simulink model "wlan_phy_rx_pmd"  (Simulink 7.8)
settings: Solver=VariableStepDiscrete  SolverMode=SingleTasking  StartTime=0.0  StopTime=simtime  RelTol=1e-3
PostLoadFcn:
  | wlan_phy_rx_init
InitFcn:
  | wlan_phy_rx_init
StartFcn:
  | wlan_phy_rx_init
values from neighbouring files (symbols this model uses):
  barker_seq20 = 0.95 * circshift(barker_seq20, [0 4]) ./ max(abs(barker_seq20))    [wlan_phy_rx_init.m]
  bit_scrambler_lfsr_bytes = bi2de(reshape(repmat(scr, 1, 8), 8, 127)', 'left-msb')    [wlan_phy_rx_init.m]
  deinterleave_ROM = [deinterleave_ROM interleave_64QAM zeros(1, 512-length(interleave_64QAM))]    [wlan_phy_rx_init.m]
  longCorr_coef_i = [3*real(LTS_corr)]    [wlan_phy_rx_init.m]
  longCorr_coef_q = [3*imag(LTS_corr)]    [wlan_phy_rx_init.m]
  MAX_CP_LEN = 32    [wlan_phy_rx_init.m]
  MAX_NCBPS = 288    [wlan_phy_rx_init.m]
  MAX_NUM_BYTES = 4096    [wlan_phy_rx_init.m]
  MAX_NUM_SC = 64    [wlan_phy_rx_init.m]
  MAX_NUM_SYMS = 600    [wlan_phy_rx_init.m]
  n_bits_preFFT_sampBuff = ceil(log2(4*MAX_NUM_SC))    [wlan_phy_rx_init.m]
  NCBPS_16QAM = 192    [wlan_phy_rx_init.m]
  NCBPS_64QAM = 288    [wlan_phy_rx_init.m]
  NCBPS_BPSK = 48    [wlan_phy_rx_init.m]
  NCBPS_QPSK = 96    [wlan_phy_rx_init.m]
  NDBPS_16QAM12 = 96    [wlan_phy_rx_init.m]
  NDBPS_16QAM34 = 144    [wlan_phy_rx_init.m]
  NDBPS_64QAM23 = 192    [wlan_phy_rx_init.m]
  NDBPS_64QAM34 = 216    [wlan_phy_rx_init.m]
  NDBPS_BPSK12 = 24    [wlan_phy_rx_init.m]
  NDBPS_BPSK34 = 36    [wlan_phy_rx_init.m]
  NDBPS_QPSK12 = 48    [wlan_phy_rx_init.m]
  NDBPS_QPSK34 = 72    [wlan_phy_rx_init.m]
  paylod_vec_samp_time = 8    [wlan_phy_rx_init.m]
  PHY_MIN_PKT_LEN = 14    [wlan_phy_rx_init.m]
  scr_ind_rev = zeros(1,128)    [wlan_phy_rx_init.m]
undefined symbols (not defined in the file or in its folder): gcbh, xlBlockLoadCallback, xlGetNormalizedPeriod, xlipgetpartsetting
libraries: xbsIndex_r4 x3119, simulink x1, xbsEDKLib_r4 x1
(part 9 of 19)

== system wlan_phy_rx_pmd/Detect & Decode/De-Interleave & De-Puncture/Rd Addr Gen ==
  note: This is a brute force de-interleaver- the ROM stores the
      | de-interleaving pattern for each mod scheme separately.
      | The 802.11 standard interleavers have enough structure that
      | a non-ROM implementation is possible. But this design will
      | enable other interleaving patterns without changes to logic.
  Block Wr Done : Inport
  mod_sel : Inport  Port=2
  Reset : Inport  Port=3
  rate_sel : Inport  Port=4
  Bus Creator : BusCreator  Inputs=2
  Bus Creator1 : BusCreator  Inputs=2
  Bus Creator2 : BusCreator  Inputs=2
  Ctrl : Subsystem
  De-Interleave DP-ROM : Subsystem
  Delay{1..4} (4 alike) : xbsIndex_r4/Delay  rst=off  infoeditControl=Selection of Reset will increase slice count due to use of real FFs and instead of SRLs  en=off  latency=1  dbl_ovrd=off  reg_retiming=off  block_type=delay
  Gateway Out{1..6,8..11} (10 alike) : xbsIndex_r4/Gateway Out  inherit_from_input=off  hdl_port=off  timing_constraint=None  locs_specified=off  LOCs={}  UseAsDAC=off  DACChannel='1'  block_type=gatewayout
  Int Rd Addr Gen : Scope  NumInputPorts=8
      settings: TimeRange=9000  YMin=0~0~0~0~-1~0~41.76470588235293~-0.1  YMax=2~1~1~1000~1~1~60.58823529411768~1.1  SaveName=ScopeData12
  Logical5 : xbsIndex_r4/Logical  logical_function=OR  inputs=2  en=off  latency=0  precision=Full  arith_type=Unsigned  n_bits=16  bin_pt=0  align_bp=on  dbl_ovrd=off  block_type=logical
  S-R Latch1 : Subsystem
  Rd Addr A : Outport
  Rd Addr B : Outport  Port=2
  Skip A : Outport  Port=3
  Skip B : Outport  Port=4
  Vout : Outport  Port=5
  wires:
  Ctrl/5(Vout) -> Delay2/1
  Gateway Out4/1 -> Int Rd Addr Gen/3  "Count En"
  Gateway Out3/1 -> Int Rd Addr Gen/2  "Wr done"
  Gateway Out2/1 -> Int Rd Addr Gen/1  "Rate Sel"
  Gateway Out8/1 -> Int Rd Addr Gen/6  "Vout"
  Logical5/1 -> S-R Latch1/2(R), Ctrl/3(Reset)
  Block Wr Done/1 -> Delay3/1
  S-R Latch1/1(Q) -> Ctrl/4(En), Gateway Out4/1
  Reset/1 -> Logical5/2
  Delay4/1 -> Skip A/1, Gateway Out9/1
  Delay3/1 -> S-R Latch1/1(S)
  Ctrl/6(Done) -> Logical5/1, Gateway Out3/1
  Delay1/1 -> Skip B/1, Gateway Out10/1
  De-Interleave DP-ROM/1(Addr A) -> Rd Addr A/1, Gateway Out6/1
  De-Interleave DP-ROM/2(Addr B) -> Rd Addr B/1, Gateway Out11/1
  Bus Creator1/1 -> Int Rd Addr Gen/7  "Rd Addr AB"
  Gateway Out6/1 -> Bus Creator1/1
  Gateway Out11/1 -> Bus Creator1/2
  mod_sel/1 -> Ctrl/1(mod_sel)
  rate_sel/1 -> Ctrl/2(rate_sel), Gateway Out2/1
  Ctrl/1(Ind A) -> De-Interleave DP-ROM/1(Ind A), Gateway Out1/1
  Ctrl/2(Ind B) -> De-Interleave DP-ROM/2(Ind B), Gateway Out5/1
  Ctrl/3(Skip A) -> Delay4/1
  Ctrl/4(Skip B) -> Delay1/1
  Delay2/1 -> Vout/1, Gateway Out8/1
  Gateway Out9/1 -> Bus Creator/1
  Gateway Out10/1 -> Bus Creator/2
  Bus Creator/1 -> Int Rd Addr Gen/8  "Skip AB"
  Bus Creator2/1 -> Int Rd Addr Gen/4  "Rd Ind AB"
  Gateway Out1/1 -> Bus Creator2/1
  Gateway Out5/1 -> Bus Creator2/2

== system wlan_phy_rx_pmd/Detect & Decode/De-Interleave & De-Puncture/Wr Addr Gen ==
  tvalid : Inport
  mod_sel : Inport  Port=2
  Reset : Inport  Port=3
  AddSub : xbsIndex_r4/AddSub  mode=Addition  use_carryin=off  use_carryout=off  en=off  latency=0  precision=User Defined  arith_type=Unsigned  n_bits=ceil(log2(MAX_NCBPS))  bin_pt=0  quantization=Truncate  overflow=Wrap  dbl_ovrd=off  use_behavioral_HDL=off  hw_selection=Fabric  pipelined=off  block_type=addsub
  Constant1 : xbsIndex_r4/Constant  const=NCBPS_QPSK-1  gui_display_data_type=Fixed-point  arith_type=Unsigned  n_bits=ceil(log2(MAX_NCBPS))  bin_pt=0  preci_type=Single  exp_width=8  frac_width=24  explicit_period=off  period=1  equ=P=C  opselect=C  inp2=PCIN>>17  opr=+  inp1=P  carry=CIN  dbl_ovrd=off  block_type=constant
  Constant3 : xbsIndex_r4/Constant  const=NCBPS_16QAM-1  gui_display_data_type=Fixed-point  arith_type=Unsigned  n_bits=ceil(log2(MAX_NCBPS))  bin_pt=0  preci_type=Single  exp_width=8  frac_width=24  explicit_period=off  period=1  equ=P=C  opselect=C  inp2=PCIN>>17  opr=+  inp1=P  carry=CIN  dbl_ovrd=off  block_type=constant
  Constant4 : xbsIndex_r4/Constant  const=NCBPS_64QAM-1  gui_display_data_type=Fixed-point  arith_type=Unsigned  n_bits=ceil(log2(MAX_NCBPS))  bin_pt=0  preci_type=Single  exp_width=8  frac_width=24  explicit_period=off  period=1  equ=P=C  opselect=C  inp2=PCIN>>17  opr=+  inp1=P  carry=CIN  dbl_ovrd=off  block_type=constant
  Constant5 : xbsIndex_r4/Constant  const=NCBPS_BPSK-1  gui_display_data_type=Fixed-point  arith_type=Unsigned  n_bits=ceil(log2(MAX_NCBPS))  bin_pt=0  preci_type=Single  exp_width=8  frac_width=24  explicit_period=off  period=1  equ=P=C  opselect=C  inp2=PCIN>>17  opr=+  inp1=P  carry=CIN  dbl_ovrd=off  block_type=constant
  Constant6 : xbsIndex_r4/Constant  const=1  gui_display_data_type=Fixed-point  arith_type=Unsigned  n_bits=1  bin_pt=0  preci_type=Single  exp_width=8  frac_width=24  explicit_period=off  period=1  equ=P=C  opselect=C  inp2=PCIN>>17  opr=+  inp1=P  carry=CIN  dbl_ovrd=off  block_type=constant
  Counter : xbsIndex_r4/Counter  cnt_type=Free Running  cnt_to=1  operation=Up  start_count=0  cnt_by_val=2  arith_type=Unsigned  n_bits=ceil(log2(MAX_NCBPS))  bin_pt=0  load_pin=off  rst=on  en=on  explicit_period=off  period=1  dbl_ovrd=off  use_behavioral_HDL=off  implementation=Fabric  block_type=counter
  DeInt Wr Addr : Scope  NumInputPorts=8
      settings: TimeRange=20000  YMin=0~0~0~0~0~-1~-1~-1  YMax=1~1~100~1~1~1~1~1  SaveName=ScopeData6
  Gateway Out{1..6} (6 alike) : xbsIndex_r4/Gateway Out  inherit_from_input=off  hdl_port=off  timing_constraint=None  locs_specified=off  LOCs={}  UseAsDAC=off  DACChannel='1'  block_type=gatewayout
  Logical5 : xbsIndex_r4/Logical  logical_function=OR  inputs=2  en=off  latency=0  precision=Full  arith_type=Unsigned  n_bits=16  bin_pt=0  align_bp=on  dbl_ovrd=off  block_type=logical
  Mux2 : xbsIndex_r4/Mux  inputs=4  en=off  latency=0  precision=Full  arith_type=Unsigned  n_bits=16  bin_pt=14  quantization=Truncate  overflow=Wrap  dbl_ovrd=off  block_type=mux
  Relational1 : xbsIndex_r4/Relational  mode=a>=b  en=off  latency=0  dbl_ovrd=off  block_type=relational
  Wr Addr A : Outport
  Wr Addr B : Outport  Port=2
  Wr En : Outport  Port=3
  Block Wr Done : Outport  Port=4
  wires:
  Mux2/1 -> Relational1/2
  Logical5/1 -> Counter/1, Gateway Out2/1
  Counter/1 -> Relational1/1, AddSub/2, Wr Addr A/1, Gateway Out4/1
  tvalid/1 -> Counter/2, Gateway Out3/1, Wr En/1, Gateway Out1/1
  Constant5/1 -> Mux2/2
  Constant1/1 -> Mux2/3
  Constant3/1 -> Mux2/4
  Constant4/1 -> Mux2/5
  mod_sel/1 -> Mux2/1
  Relational1/1 -> Gateway Out5/1, Block Wr Done/1, Logical5/2
  Reset/1 -> Logical5/1
  Gateway Out2/1 -> DeInt Wr Addr/1  "Reset"
  Gateway Out3/1 -> DeInt Wr Addr/2  "Valid In"
  Gateway Out4/1 -> DeInt Wr Addr/3  "Wr Addr A"
  Gateway Out1/1 -> DeInt Wr Addr/5  "Wr En"
  Gateway Out5/1 -> DeInt Wr Addr/6  "Block Done"
  Constant6/1 -> AddSub/1
  AddSub/1 -> Wr Addr B/1, Gateway Out6/1
  Gateway Out6/1 -> DeInt Wr Addr/4  "Wr Addr B"

== system wlan_phy_rx_pmd/Detect & Decode/Decode/Byte Count ==
  Byte Valid : Inport
  Decode Start : Inport  Port=2
  Last Byte Ind : Inport  Port=3
  Byte Counter : xbsIndex_r4/Counter  cnt_type=Free Running  cnt_to=1  operation=Up  start_count=0  cnt_by_val=1  arith_type=Unsigned  n_bits=14  bin_pt=0  load_pin=off  rst=on  en=on  explicit_period=off  period=1  dbl_ovrd=off  use_behavioral_HDL=off  implementation=Fabric  block_type=counter
  Inverter1 : xbsIndex_r4/Inverter  en=off  latency=0  dbl_ovrd=off  block_type=inv
  Logical : xbsIndex_r4/Logical  logical_function=AND  inputs=2  en=off  latency=0  precision=Full  arith_type=Unsigned  n_bits=16  bin_pt=0  align_bp=on  dbl_ovrd=off  block_type=logical
  Logical1 : xbsIndex_r4/Logical  logical_function=OR  inputs=2  en=off  latency=0  precision=Full  arith_type=Unsigned  n_bits=16  bin_pt=0  align_bp=on  dbl_ovrd=off  block_type=logical
  Logical3 : xbsIndex_r4/Logical  logical_function=AND  inputs=2  en=off  latency=0  precision=Full  arith_type=Unsigned  n_bits=16  bin_pt=0  align_bp=on  dbl_ovrd=off  block_type=logical
  Relational2 : xbsIndex_r4/Relational  mode=a>=b  en=off  latency=0  dbl_ovrd=off  block_type=relational
  S-R Latch1 : Subsystem
  " Byte Valid" : Outport
  Byte Index : Outport  Port=2
  Last Byte : Outport  Port=3
  wires:
  Logical/1 -> Byte Counter/2, " Byte Valid"/1
  Byte Counter/1 -> Byte Index/1, Relational2/1
  Logical3/1 -> S-R Latch1/2(R), Last Byte/1
  Decode Start/1 -> S-R Latch1/1(S), Logical1/1
  S-R Latch1/1(Q) -> Logical/1, Inverter1/1
  Byte Valid/1 -> Logical/2, Logical3/1
  Relational2/1 -> Logical3/2
  Last Byte Ind/1 -> Relational2/2
  Inverter1/1 -> Logical1/2
  Logical1/1 -> Byte Counter/1

== system wlan_phy_rx_pmd/Detect & Decode/Decode/Ctrl ==
  note: early_trace input requires pulse coincident
      | with last vin for early bits, staying asserted
      | until at least first decoder vout.
  note: 22: (NUM_SIGNAL_BYTES * 8) - 2
  dec_vin : Inport
  Reset : Inport  Port=2
  Start : Inport  Port=3
  Num Bytes : Inport  Port=4
  dec_vout : Inport  Port=5
  AddSub : xbsIndex_r4/AddSub  mode=Addition  use_carryin=off  use_carryout=off  en=off  latency=0  precision=Full  arith_type=Unsigned  n_bits=16  bin_pt=14  quantization=Truncate  overflow=Wrap  dbl_ovrd=off  use_behavioral_HDL=off  hw_selection=Fabric  pipelined=off  block_type=addsub
  Coded Bit Counter : xbsIndex_r4/Counter  cnt_type=Free Running  cnt_to=1  operation=Up  start_count=0  cnt_by_val=1  arith_type=Unsigned  n_bits=2+ceil(log2(MAX_NUM_BYTES))  bin_pt=0  load_pin=off  rst=on  en=on  explicit_period=off  period=1  dbl_ovrd=off  use_behavioral_HDL=off  implementation=Fabric  block_type=counter
  Concat : xbsIndex_r4/Concat  num_inputs=2  dbl_ovrd=off  block_type=concat
  Constant1 : xbsIndex_r4/Constant  const=14  gui_display_data_type=Fixed-point  arith_type=Unsigned  n_bits=5  bin_pt=0  preci_type=Single  exp_width=8  frac_width=24  explicit_period=off  period=1  equ=P=C  opselect=C  inp2=PCIN>>17  opr=+  inp1=P  carry=CIN  dbl_ovrd=off  block_type=constant
  Constant2 : xbsIndex_r4/Constant  const=0  gui_display_data_type=Fixed-point  arith_type=Unsigned  n_bits=3  bin_pt=0  preci_type=Single  exp_width=8  frac_width=24  explicit_period=off  period=1  equ=P=C  opselect=C  inp2=PCIN>>17  opr=+  inp1=P  carry=CIN  dbl_ovrd=off  block_type=constant
  Constant3 : xbsIndex_r4/Constant  const=3*8 - 2  gui_display_data_type=Fixed-point  arith_type=Unsigned  n_bits=5  bin_pt=0  preci_type=Single  exp_width=8  frac_width=24  explicit_period=off  period=1  equ=P=C  opselect=C  inp2=PCIN>>17  opr=+  inp1=P  carry=CIN  dbl_ovrd=off  block_type=constant
  Convert{,1..2,7} (4 alike) : xbsIndex_r4/Convert  gui_display_data_type=Fixed-point  arith_type=Unsigned  n_bits=1  bin_pt=0  float_type=Single  exp_bits=8  fraction_bits=24  quantization=Truncate  overflow=Wrap  en=off  latency=0  dbl_ovrd=off  pipeline=off  block_type=convert
  Decoder Ctrl : Scope  NumInputPorts=8
      settings: TimeRange=10000  YMin=0~0~0~0~0~0~0~0  YMax=1~1~100~1~1000~1~1~1  SaveName=ScopeData40
  Delay1 : xbsIndex_r4/Delay  rst=off  infoeditControl=Selection of Reset will increase slice count due to use of real FFs and instead of SRLs  en=off  latency=2  dbl_ovrd=off  reg_retiming=off  block_type=delay
  Delay2 : xbsIndex_r4/Delay  rst=off  infoeditControl=Selection of Reset will increase slice count due to use of real FFs and instead of SRLs  en=off  latency=2  dbl_ovrd=off  reg_retiming=off  block_type=delay
  Gateway Out{1..5,7..8,10} (8 alike) : xbsIndex_r4/Gateway Out  inherit_from_input=off  hdl_port=off  timing_constraint=None  locs_specified=off  LOCs={}  UseAsDAC=off  DACChannel='1'  block_type=gatewayout
  Inverter : xbsIndex_r4/Inverter  en=off  latency=0  dbl_ovrd=off  block_type=inv
  Logical1 : xbsIndex_r4/Logical  logical_function=AND  inputs=2  en=off  latency=0  precision=Full  arith_type=Unsigned  n_bits=16  bin_pt=0  align_bp=on  dbl_ovrd=off  block_type=logical
  Logical3 : xbsIndex_r4/Logical  logical_function=OR  inputs=2  en=off  latency=0  precision=Full  arith_type=Unsigned  n_bits=16  bin_pt=0  align_bp=on  dbl_ovrd=off  block_type=logical
  Relational2 : xbsIndex_r4/Relational  mode=a<b  en=off  latency=0  dbl_ovrd=off  block_type=relational
  Relational3 : xbsIndex_r4/Relational  mode=a=b  en=off  latency=0  dbl_ovrd=off  block_type=relational
  S-R Latch1 : Subsystem
  negedge : Subsystem
  vin : Outport
  rst# : Outport  Port=2
  start : Outport  Port=3
  pkt_end : Outport  Port=4
  early_trace_en : Outport  Port=5
  wires:
  dec_vin/1 -> Coded Bit Counter/2, Gateway Out3/1, Logical1/2
  dec_vout/1 -> S-R Latch1/2(R)
  S-R Latch1/1(Q) -> Convert1/1
  Start/1 -> Coded Bit Counter/1, Gateway Out1/1, Logical3/2, Delay1/1
  Coded Bit Counter/1 -> Gateway Out4/1, Relational3/2, Relational2/1
  Num Bytes/1 -> Concat/1, Gateway Out2/1
  Constant2/1 -> Concat/2
  Concat/1 -> AddSub/1
  Inverter/1 -> Convert/1
  Logical3/1 -> Inverter/1
  Reset/1 -> Logical3/1, Gateway Out10/1
  Delay1/1 -> Convert7/1
  Convert/1 -> rst#/1
  Convert7/1 -> start/1
  Relational3/1 -> S-R Latch1/1(S)
  Convert1/1 -> early_trace_en/1, Gateway Out5/1
  Gateway Out10/1 -> Decoder Ctrl/1  "Reset"
  Gateway Out1/1 -> Decoder Ctrl/2  "Start"
  Gateway Out2/1 -> Decoder Ctrl/3  "Num Bytes"
  Gateway Out3/1 -> Decoder Ctrl/4  "Vin in"
  Gateway Out4/1 -> Decoder Ctrl/5  "Bit Counter"
  Gateway Out5/1 -> Decoder Ctrl/6  "Early Trace En"
  negedge/1(Q) -> Delay2/1
  Gateway Out7/1 -> Decoder Ctrl/7  "End"
  Constant3/1 -> Relational3/1
  Logical1/1 -> Convert2/1
  Convert2/1 -> vin/1, Gateway Out8/1
  Gateway Out8/1 -> Decoder Ctrl/8  "Vin Out"
  Relational2/1 -> Logical1/1, negedge/1(D)
  Constant1/1 -> AddSub/2
  AddSub/1 -> Relational2/2
  Delay2/1 -> pkt_end/1, Gateway Out7/1

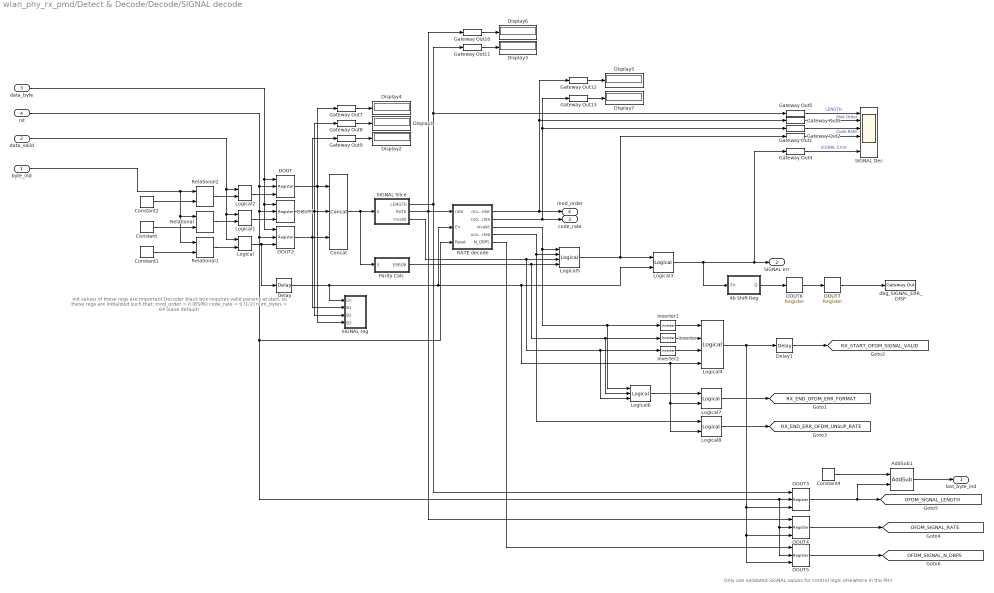
== system wlan_phy_rx_pmd/Detect & Decode/Decode/SIGNAL decode  ==
  note: Init values of these regs are important
      | Decoder black box requires valid params at start,
      | so these regs are initialzied such that:
      | mod_order = 0 (BSPK)
      | code_rate = 0 (1/2)
      | num_bytes = 64 (sane default)
  note: Only use validated SIGNAL values for
      | control logic elsewhere in the PHY
  byte_ind : Inport
  data_valid : Inport  Port=2
  data_byte : Inport  Port=3
  rst : Inport  Port=4
  4b Shift Reg : Subsystem
  AddSub1 : xbsIndex_r4/AddSub  mode=Addition  use_carryin=off  use_carryout=off  en=off  latency=0  precision=User Defined  arith_type=Unsigned  n_bits=12  bin_pt=0  quantization=Truncate  overflow=Wrap  dbl_ovrd=off  use_behavioral_HDL=off  hw_selection=Fabric  pipelined=off  block_type=addsub
  Concat : xbsIndex_r4/Concat  num_inputs=3  dbl_ovrd=off  block_type=concat
  Constant : xbsIndex_r4/Constant  const=1  gui_display_data_type=Fixed-point  arith_type=Unsigned  n_bits=2  bin_pt=0  preci_type=Single  exp_width=8  frac_width=24  explicit_period=off  period=1  equ=P=C  opselect=C  inp2=PCIN>>17  opr=+  inp1=P  carry=CIN  dbl_ovrd=off  block_type=constant
  Constant1 : xbsIndex_r4/Constant  const=2  gui_display_data_type=Fixed-point  arith_type=Unsigned  n_bits=2  bin_pt=0  preci_type=Single  exp_width=8  frac_width=24  explicit_period=off  period=1  equ=P=C  opselect=C  inp2=PCIN>>17  opr=+  inp1=P  carry=CIN  dbl_ovrd=off  block_type=constant
  Constant2 : xbsIndex_r4/Constant  const=0  gui_display_data_type=Fixed-point  arith_type=Unsigned  n_bits=2  bin_pt=0  preci_type=Single  exp_width=8  frac_width=24  explicit_period=off  period=1  equ=P=C  opselect=C  inp2=PCIN>>17  opr=+  inp1=P  carry=CIN  dbl_ovrd=off  block_type=constant
  Constant4 : xbsIndex_r4/Constant  const=4  gui_display_data_type=Fixed-point  arith_type=Unsigned  n_bits=3  bin_pt=0  preci_type=Single  exp_width=8  frac_width=24  explicit_period=off  period=1  equ=P=C  opselect=C  inp2=PCIN>>17  opr=+  inp1=P  carry=CIN  dbl_ovrd=off  block_type=constant
  DOUT : xbsIndex_r4/Register  init=hex2dec('D6')  rst=on  en=on  dbl_ovrd=off  block_type=register
  DOUT1 : xbsIndex_r4/Register  init=hex2dec('E0')  rst=on  en=on  dbl_ovrd=off  block_type=register
  DOUT2 : xbsIndex_r4/Register  init=hex2dec('00')  rst=on  en=on  dbl_ovrd=off  block_type=register
  DOUT3 : xbsIndex_r4/Register  init=10  rst=on  en=on  dbl_ovrd=off  block_type=register
  DOUT4 : xbsIndex_r4/Register  init=11  rst=on  en=on  dbl_ovrd=off  block_type=register
  DOUT5 : xbsIndex_r4/Register  init=24  rst=on  en=on  dbl_ovrd=off  block_type=register
  DOUT6 : xbsIndex_r4/Register  init=0  rst=off  en=off  dbl_ovrd=off  block_type=register
  DOUT7 : xbsIndex_r4/Register  init=0  rst=off  en=off  dbl_ovrd=off  block_type=register
  Delay : xbsIndex_r4/Delay  rst=off  infoeditControl=Selection of Reset will increase slice count due to use of real FFs and instead of SRLs  en=off  latency=1  dbl_ovrd=off  reg_retiming=off  block_type=delay
  Delay1 : xbsIndex_r4/Delay  rst=off  infoeditControl=Selection of Reset will increase slice count due to use of real FFs and instead of SRLs  en=off  latency=1  dbl_ovrd=off  reg_retiming=off  block_type=delay
  Display{1..7} (7 alike) : Display
  Gateway Out{1..2,4..13} (12 alike) : xbsIndex_r4/Gateway Out  inherit_from_input=off  hdl_port=off  timing_constraint=None  locs_specified=off  LOCs={}  UseAsDAC=off  DACChannel='1'  block_type=gatewayout
  Inverter : xbsIndex_r4/Inverter  en=off  latency=0  dbl_ovrd=off  block_type=inv
  Inverter1 : xbsIndex_r4/Inverter  en=off  latency=0  dbl_ovrd=off  block_type=inv
  Inverter2 : xbsIndex_r4/Inverter  en=off  latency=0  dbl_ovrd=off  block_type=inv
  Logical{,1..3,7..8} (6 alike) : xbsIndex_r4/Logical  logical_function=AND  inputs=2  en=off  latency=0  precision=Full  arith_type=Unsigned  n_bits=16  bin_pt=0  align_bp=on  dbl_ovrd=off  block_type=logical
  Logical4 : xbsIndex_r4/Logical  logical_function=AND  inputs=4  en=off  latency=0  precision=Full  arith_type=Unsigned  n_bits=16  bin_pt=0  align_bp=on  dbl_ovrd=off  block_type=logical
  Logical5 : xbsIndex_r4/Logical  logical_function=OR  inputs=4  en=off  latency=0  precision=Full  arith_type=Unsigned  n_bits=16  bin_pt=0  align_bp=on  dbl_ovrd=off  block_type=logical
  Logical6 : xbsIndex_r4/Logical  logical_function=OR  inputs=3  en=off  latency=0  precision=Full  arith_type=Unsigned  n_bits=16  bin_pt=0  align_bp=on  dbl_ovrd=off  block_type=logical
  Parity Calc : Subsystem
  RATE decode : Subsystem
  Relational : xbsIndex_r4/Relational  mode=a=b  en=off  latency=0  dbl_ovrd=off  block_type=relational
  Relational1 : xbsIndex_r4/Relational  mode=a=b  en=off  latency=0  dbl_ovrd=off  block_type=relational
  Relational2 : xbsIndex_r4/Relational  mode=a=b  en=off  latency=0  dbl_ovrd=off  block_type=relational
  SIGNAL Dec : Scope  NumInputPorts=6
      settings: TimeRange=25000  YMin=60.8~0.95~-1~0.95~-1~-1  YMax=67.2~1.05~1~1.05~1~1  SaveName=ScopeData18
  SIGNAL Slice : Subsystem
  SIGNAL reg : Subsystem
  dbg_SIGNAL_ERR_DISP : xbsIndex_r4/Gateway Out  inherit_from_input=off  hdl_port=on  timing_constraint=None  locs_specified=off  LOCs={}  UseAsDAC=off  DACChannel='1'  block_type=gatewayout
  last_byte_ind : Outport
  SIGNAL err : Outport  Port=2
  code_rate : Outport  Port=3
  mod_order : Outport  Port=4
  tag scope: OFDM_SIGNAL_LENGTH=global, OFDM_SIGNAL_N_DBPS=global, OFDM_SIGNAL_RATE=global, RX_END_ERR_OFDM_UNSUP_RATE=global, RX_END_OFDM_ERR_FORMAT=global, RX_START_OFDM_SIGNAL_VALID=global
  wires:
  data_valid/1 -> Logical2/1, Logical1/1, Logical/1
  byte_ind/1 -> Relational2/1, Relational1/1, Relational/1
  data_byte/1 -> DOUT/1, DOUT1/1, DOUT2/1
  Gateway Out5/1 -> SIGNAL Dec/1  "LENGTH"
  Gateway Out6/1 -> SIGNAL Dec/2  "Mod Order"
  Gateway Out1/1 -> SIGNAL Dec/3  "Code Rate"
  Gateway Out2/1 -> SIGNAL Dec/4
  Gateway Out4/1 -> SIGNAL Dec/6  "SIGNAL Error"
  Constant/1 -> Relational/2
  Constant1/1 -> Relational1/2
  Constant2/1 -> Relational2/2
  Relational1/1 -> Logical/2
  Relational2/1 -> Logical2/2
  Relational/1 -> Logical1/2
  Logical/1 -> Delay/1, DOUT2/3
  Concat/1 -> SIGNAL Slice/1(S), Parity Calc/1(S)
  SIGNAL Slice/2(RATE) -> Gateway Out10/1, DOUT4/1, RATE decode/1(rate)
  SIGNAL Slice/1(LENGTH) -> DOUT3/1, Gateway Out11/1, Gateway Out5/1
  Parity Calc/1(ERROR) -> Logical5/4, Inverter/1, Logical6/2
  Logical5/1 -> Logical3/1, Gateway Out2/1
  DOUT/1 -> Concat/1, Gateway Out7/1, SIGNAL reg/4(B3)
  DOUT1/1 -> Concat/2, Gateway Out8/1, SIGNAL reg/3(B2)
  DOUT2/1 -> Concat/3, Gateway Out9/1, SIGNAL reg/2(B1)
  Logical2/1 -> DOUT/3
  Logical1/1 -> DOUT1/3
  rst/1 -> DOUT/2, DOUT1/2, DOUT2/2, DOUT3/2, DOUT4/2, DOUT5/2, RATE decode/3(Reset)
  RATE decode/1(mod_order) -> mod_order/1, Gateway Out6/1, Gateway Out12/1
  RATE decode/2(code_rate) -> code_rate/1, Gateway Out1/1, Gateway Out13/1
  Delay/1 -> SIGNAL reg/1(En), Logical3/2, Logical4/4, Logical7/2, Logical8/2, RATE decode/2(En)
  Gateway Out7/1 -> Display4/1
  Gateway Out8/1 -> Display1/1
  Gateway Out9/1 -> Display2/1
  Gateway Out10/1 -> Display6/1
  Gateway Out11/1 -> Display3/1
  Gateway Out12/1 -> Display5/1
  Gateway Out13/1 -> Display7/1
  Logical3/1 -> SIGNAL err/1, Gateway Out4/1, 4b Shift Reg/1(En)
  Inverter/1 -> Logical4/2
  Logical4/1 -> Delay1/1, DOUT3/3, DOUT5/3, DOUT4/3
  SIGNAL Slice/3(invalid) -> Logical5/3, Inverter2/1, Logical6/3
  RATE decode/4(unsupported) -> Logical5/2, Logical8/1
  RATE decode/3(invalid) -> Logical5/1, Inverter1/1, Logical6/1
  Inverter1/1 -> Logical4/1
  Inverter2/1 -> Logical4/3
  Logical6/1 -> Logical7/1
  Logical7/1 -> [RX_END_OFDM_ERR_FORMAT]
  Logical8/1 -> [RX_END_ERR_OFDM_UNSUP_RATE]
  DOUT4/1 -> [OFDM_SIGNAL_RATE]
  DOUT3/1 -> [OFDM_SIGNAL_LENGTH], AddSub1/2
  Delay1/1 -> [RX_START_OFDM_SIGNAL_VALID]
  AddSub1/1 -> last_byte_ind/1
  RATE decode/5(N_DBPS) -> DOUT5/1
  DOUT5/1 -> [OFDM_SIGNAL_N_DBPS]
  Constant4/1 -> AddSub1/1
  DOUT6/1 -> DOUT7/1
  DOUT7/1 -> dbg_SIGNAL_ERR_DISP/1
  4b Shift Reg/1(Q) -> DOUT6/1

== system wlan_phy_rx_pmd/Detect & Decode/Decode/Safe ToUint8 ==
  note: Simulation-only block: Sets output to 0
      | if input is indeterminate, which occurs at
      | the blackbox outputs before the first reset
  A : Inport
  Convert1 : xbsIndex_r4/Convert  gui_display_data_type=Fixed-point  arith_type=Unsigned  n_bits=8  bin_pt=0  float_type=Single  exp_bits=8  fraction_bits=24  quantization=Truncate  overflow=Wrap  en=off  latency=0  dbl_ovrd=off  pipeline=off  block_type=convert
  Indet Check : Subsystem
  Simulation Multiplexer : xbsIndex_r4/Simulation Multiplexer  sim_sel=1  hw_sel=2  block_type=simmux
      settings: infoedit="Distinguish input subsystems as \"simulation only\" and \"generation only\".  The input specified For Simulation will be used during Simulink simulation.  The input specified For Generation will be used during code generation.  This block will typically be used on the output of a HW Co-Simulation, Black Box, or ModelSim subsystem.<br><br>Hardware Notes: This block costs nothing."
  B : Outport
  wires:
  A/1 -> Indet Check/1(In1), Simulation Multiplexer/2
  Indet Check/1(Out1) -> Simulation Multiplexer/1
  Simulation Multiplexer/1 -> Convert1/1
  Convert1/1 -> B/1

== system wlan_phy_rx_pmd/Detect & Decode/Decode/Stretch ==
  D : Inport
  Delay1 : xbsIndex_r4/Delay  rst=off  infoeditControl=Selection of Reset will increase slice count due to use of real FFs and instead of SRLs  en=off  latency=1  dbl_ovrd=off  reg_retiming=off  block_type=delay
  Delay4 : xbsIndex_r4/Delay  rst=off  infoeditControl=Selection of Reset will increase slice count due to use of real FFs and instead of SRLs  en=off  latency=1  dbl_ovrd=off  reg_retiming=off  block_type=delay
  Delay5 : xbsIndex_r4/Delay  rst=off  infoeditControl=Selection of Reset will increase slice count due to use of real FFs and instead of SRLs  en=off  latency=1  dbl_ovrd=off  reg_retiming=off  block_type=delay
  Logical4 : xbsIndex_r4/Logical  logical_function=OR  inputs=4  en=off  latency=0  precision=Full  arith_type=Unsigned  n_bits=16  bin_pt=0  align_bp=on  dbl_ovrd=off  block_type=logical
  Q : Outport
  wires:
  D/1 -> Logical4/1, Delay1/1
  Delay1/1 -> Logical4/2, Delay4/1
  Delay4/1 -> Logical4/3, Delay5/1
  Delay5/1 -> Logical4/4
  Logical4/1 -> Q/1

== system wlan_phy_rx_pmd/Detect & Decode/Descramble/Descram Byte Calc ==
  note: SERVICE field is used to lookup initial state
      | of Tx scrambler. This state becomes the starting
      | index to the bytewise descrambler logic. This
      | block is a parallel version of the bitwise scrambling
      | specified in the 802.11 spec.
  note: Don't keep the reset signal asserted if a packet
      | happens to end on byte 126 when the next
      | SERVICE field arrives. Without this not/and
      | every 126th packet will be descrambled wrong
      | (when all pkts have the same length)
  data_byte : Inport
  data_valid : Inport  Port=2
  data_ind : Inport  Port=3
  3LSB1 : xbsIndex_r4/Slice  nbits=3  boolean_output=off  mode=Lower Bit Location + Width  bit1=0  base1=MSB of Input  bit0=0  base0=LSB of Input  dbl_ovrd=off  block_type=slice
  4MSB1 : xbsIndex_r4/Slice  nbits=4  boolean_output=off  mode=Upper Bit Location + Width  bit1=0  base1=MSB of Input  bit0=0  base0=LSB of Input  dbl_ovrd=off  block_type=slice
  Concat1 : xbsIndex_r4/Concat  num_inputs=2  dbl_ovrd=off  block_type=concat
  Constant : xbsIndex_r4/Constant  const=3  gui_display_data_type=Fixed-point  arith_type=Unsigned  n_bits=4  bin_pt=0  preci_type=Single  exp_width=8  frac_width=24  explicit_period=off  period=1  equ=P=C  opselect=C  inp2=PCIN>>17  opr=+  inp1=P  carry=CIN  dbl_ovrd=off  block_type=constant
  Constant1 : xbsIndex_r4/Constant  const=126  gui_display_data_type=Fixed-point  arith_type=Unsigned  n_bits=7  bin_pt=0  preci_type=Single  exp_width=8  frac_width=24  explicit_period=off  period=1  equ=P=C  opselect=C  inp2=PCIN>>17  opr=+  inp1=P  carry=CIN  dbl_ovrd=off  block_type=constant
  Constant2 : xbsIndex_r4/Constant  const=0  gui_display_data_type=Fixed-point  arith_type=Unsigned  n_bits=8  bin_pt=0  preci_type=Single  exp_width=8  frac_width=24  explicit_period=off  period=1  equ=P=C  opselect=C  inp2=PCIN>>17  opr=+  inp1=P  carry=CIN  dbl_ovrd=off  block_type=constant
  Counter : xbsIndex_r4/Counter  cnt_type=Free Running  cnt_to=126  operation=Up  start_count=0  cnt_by_val=1  arith_type=Unsigned  n_bits=7  bin_pt=0  load_pin=on  rst=on  en=on  explicit_period=off  period=1  dbl_ovrd=off  use_behavioral_HDL=off  implementation=Fabric  block_type=counter
  Delay{1..4} (4 alike) : xbsIndex_r4/Delay  rst=off  infoeditControl=Selection of Reset will increase slice count due to use of real FFs and instead of SRLs  en=off  latency=1  dbl_ovrd=off  reg_retiming=off  block_type=delay
  Delay5 : xbsIndex_r4/Delay  rst=off  infoeditControl=Selection of Reset will increase slice count due to use of real FFs and instead of SRLs  en=off  latency=3  dbl_ovrd=off  reg_retiming=off  block_type=delay
  Descrm Ind : Scope  NumInputPorts=8
      settings: TimeRange=8000  YMin=70~102~50~-1~-5~-5~-5~-5  YMax=210~105~135~1~5~5~5~5  SaveName=ScopeData19
  Gateway Out{1..8} (8 alike) : xbsIndex_r4/Gateway Out  inherit_from_input=off  hdl_port=off  timing_constraint=None  locs_specified=off  LOCs={}  UseAsDAC=off  DACChannel='1'  block_type=gatewayout
  Inverter : xbsIndex_r4/Inverter  en=off  latency=0  dbl_ovrd=off  block_type=inv
  LFSR Init : Subsystem
  LFSR Init State LUT : xbsIndex_r4/ROM  depth=length(scr_ind_rev)  initVector=scr_ind_rev  distributed_mem=Distributed memory  rst=off  init_reg=0  en=off  latency=0  gui_display_data_type=Fixed-point  arith_type=Unsigned  n_bits=7  bin_pt=0  preci_type=Single  exp_width=8  frac_width=24  dbl_ovrd=off  optimize=Area  use_rpm=on  block_type=sprom
  LFSR by Byte : xbsIndex_r4/ROM  depth=length(bit_scrambler_lfsr_bytes)  initVector=bit_scrambler_lfsr_bytes  distributed_mem=Block RAM  rst=off  init_reg=0  en=off  latency=1  gui_display_data_type=Fixed-point  arith_type=Unsigned  n_bits=8  bin_pt=0  preci_type=Single  exp_width=8  frac_width=24  dbl_ovrd=off  optimize=Area  use_rpm=on  block_type=sprom
  Logical : xbsIndex_r4/Logical  logical_function=AND  inputs=2  en=off  latency=0  precision=Full  arith_type=Unsigned  n_bits=16  bin_pt=0  align_bp=on  dbl_ovrd=off  block_type=logical
  Logical1 : xbsIndex_r4/Logical  logical_function=OR  inputs=2  en=off  latency=0  precision=Full  arith_type=Unsigned  n_bits=16  bin_pt=0  align_bp=on  dbl_ovrd=off  block_type=logical
  Logical2 : xbsIndex_r4/Logical  logical_function=AND  inputs=3  en=off  latency=0  precision=Full  arith_type=Unsigned  n_bits=16  bin_pt=0  align_bp=on  dbl_ovrd=off  block_type=logical
  Mux : xbsIndex_r4/Mux  inputs=2  en=off  latency=0  precision=Full  arith_type=Unsigned  n_bits=16  bin_pt=14  quantization=Truncate  overflow=Wrap  dbl_ovrd=off  block_type=mux
  Relational : xbsIndex_r4/Relational  mode=a=b  en=off  latency=0  dbl_ovrd=off  block_type=relational
  Relational1 : xbsIndex_r4/Relational  mode=a=b  en=off  latency=0  dbl_ovrd=off  block_type=relational
  Relational2 : xbsIndex_r4/Relational  mode=a>=b  en=off  latency=0  dbl_ovrd=off  block_type=relational
  SERVICE : xbsIndex_r4/Register  init=0  rst=off  en=on  dbl_ovrd=off  block_type=register
  Descram Val : Outport
  wires:
  Logical2/1 -> Counter/3
  Relational1/1 -> Logical2/1
  Delay4/1 -> Gateway Out3/1
  Delay2/1 -> Delay4/1
  Concat1/1 -> Gateway Out1/1, Counter/2
  Gateway Out4/1 -> Descrm Ind/6
  4MSB1/1 -> Concat1/2
  3LSB1/1 -> Concat1/1
  Gateway Out3/1 -> Descrm Ind/5
  Gateway Out2/1 -> Descrm Ind/4
  Gateway Out1/1 -> Descrm Ind/3
  Gateway Out6/1 -> Descrm Ind/2
  Gateway Out5/1 -> Descrm Ind/1
  Counter/1 -> LFSR by Byte/1, Gateway Out2/1, Relational1/1
  Constant1/1 -> Relational1/2
  Delay1/1 -> Logical2/2, Logical1/2, Delay2/1
  Logical1/1 -> Counter/4
  Delay3/1 -> Logical1/1, Counter/1, Inverter/1
  LFSR Init State LUT/1 -> 4MSB1/1, 3LSB1/1, Gateway Out6/1
  LFSR by Byte/1 -> Gateway Out4/1, Mux/3
  data_ind/1 -> Relational/1, Relational2/1, Gateway Out8/1
  data_valid/1 -> Delay1/1, Logical/1
  data_byte/1 -> SERVICE/1
  LFSR Init/1(I) -> Gateway Out5/1, LFSR Init State LUT/1
  SERVICE/1 -> LFSR Init/1(B)
  Logical/1 -> Delay3/1, SERVICE/2
  Relational/1 -> Logical/2
  Constant/1 -> Relational/2, Relational2/2
  Mux/1 -> Descram Val/1
  Relational2/1 -> Delay5/1
  Constant2/1 -> Mux/2
  Gateway Out7/1 -> Descrm Ind/7
  Gateway Out8/1 -> Descrm Ind/8
  Delay5/1 -> Mux/1, Gateway Out7/1
  Inverter/1 -> Logical2/3

== system wlan_phy_rx_pmd/Detect & Decode/Descramble/Endian Swap ==
  B : Inport
  Concat : xbsIndex_r4/Concat  num_inputs=8  dbl_ovrd=off  block_type=concat
  b0 : xbsIndex_r4/Slice  nbits=1  boolean_output=off  mode=Lower Bit Location + Width  bit1=0  base1=MSB of Input  bit0=0  base0=LSB of Input  dbl_ovrd=off  block_type=slice
  b1 : xbsIndex_r4/Slice  nbits=1  boolean_output=off  mode=Lower Bit Location + Width  bit1=0  base1=MSB of Input  bit0=1  base0=LSB of Input  dbl_ovrd=off  block_type=slice
  b2 : xbsIndex_r4/Slice  nbits=1  boolean_output=off  mode=Lower Bit Location + Width  bit1=0  base1=MSB of Input  bit0=2  base0=LSB of Input  dbl_ovrd=off  block_type=slice
  b3 : xbsIndex_r4/Slice  nbits=1  boolean_output=off  mode=Lower Bit Location + Width  bit1=0  base1=MSB of Input  bit0=3  base0=LSB of Input  dbl_ovrd=off  block_type=slice
  b4 : xbsIndex_r4/Slice  nbits=1  boolean_output=off  mode=Lower Bit Location + Width  bit1=0  base1=MSB of Input  bit0=4  base0=LSB of Input  dbl_ovrd=off  block_type=slice
  b5 : xbsIndex_r4/Slice  nbits=1  boolean_output=off  mode=Lower Bit Location + Width  bit1=0  base1=MSB of Input  bit0=5  base0=LSB of Input  dbl_ovrd=off  block_type=slice
  b6 : xbsIndex_r4/Slice  nbits=1  boolean_output=off  mode=Lower Bit Location + Width  bit1=0  base1=MSB of Input  bit0=6  base0=LSB of Input  dbl_ovrd=off  block_type=slice
  b7 : xbsIndex_r4/Slice  nbits=1  boolean_output=off  mode=Lower Bit Location + Width  bit1=0  base1=MSB of Input  bit0=7  base0=LSB of Input  dbl_ovrd=off  block_type=slice
  I : Outport
  wires:
  b7/1 -> Concat/8
  Concat/1 -> I/1
  b6/1 -> Concat/7
  b5/1 -> Concat/6
  b4/1 -> Concat/5
  b3/1 -> Concat/4
  b2/1 -> Concat/3
  b1/1 -> Concat/2
  b0/1 -> Concat/1
  B/1 -> b7/1, b6/1, b5/1, b4/1, b3/1, b2/1, b1/1, b0/1

== system wlan_phy_rx_pmd/Detect & Decode/Soft Demap/16QAM Soft De-Map ==
  note: 16-QAM Demap:
      | Each sym is [b0 b1 b2 b3]
      | b0 = 1 if (I > 0)
      | b1 = 1 if(abs(I) < 2/sqrt(10))
      | b2 = 1 if(Q > 0)
      | b3 = 1 if(abs(Q) < 2/sqrt(10))
  IQ Valid : Inport
  I : Inport  Port=2
  Q : Inport  Port=3
  Reest : Inport  Port=4
  Absolute : xbsIndex_r4/Absolute  precision=Full  arith_type=Unsigned  n_bits=8  bin_pt=0  quantization=Truncate  overflow=Saturate  en=off  latency=0  dbl_ovrd=off  block_type=abs
  AddSub : xbsIndex_r4/AddSub  mode=Subtraction  use_carryin=off  use_carryout=off  en=off  latency=0  precision=User Defined  arith_type="Signed  (2's comp)"  n_bits=8  bin_pt=7  quantization=Truncate  overflow=Saturate  dbl_ovrd=off  use_behavioral_HDL=off  hw_selection=Fabric  pipelined=off  block_type=addsub
  Constant : xbsIndex_r4/Constant  const=2/sqrt(10)  gui_display_data_type=Fixed-point  arith_type=Unsigned  n_bits=12  bin_pt=12  preci_type=Single  exp_width=8  frac_width=24  explicit_period=off  period=1  equ=P=C  opselect=C  inp2=PCIN>>17  opr=+  inp1=P  carry=CIN  dbl_ovrd=off  block_type=constant
  Delay2 : xbsIndex_r4/Delay  rst=off  infoeditControl=Selection of Reset will increase slice count due to use of real FFs and instead of SRLs  en=off  latency=1  dbl_ovrd=off  reg_retiming=off  block_type=delay
  Inverter : xbsIndex_r4/Inverter  en=off  latency=0  dbl_ovrd=off  block_type=inv
      settings: xl_area=[0 0 0 0 0 0 0]  block_version=VER_STRING_GOES_HERE
  Logical1 : xbsIndex_r4/Logical  logical_function=OR  inputs=2  en=off  latency=0  precision=Full  arith_type=Unsigned  n_bits=16  bin_pt=0  align_bp=on  dbl_ovrd=off  block_type=logical
  Logical2 : xbsIndex_r4/Logical  logical_function=OR  inputs=2  en=off  latency=0  precision=Full  arith_type=Unsigned  n_bits=16  bin_pt=0  align_bp=on  dbl_ovrd=off  block_type=logical
  Mux : xbsIndex_r4/Mux  inputs=2  en=off  latency=0  precision=Full  arith_type=Unsigned  n_bits=16  bin_pt=14  quantization=Truncate  overflow=Wrap  dbl_ovrd=off  block_type=mux
  Register1 : xbsIndex_r4/Register  init=0  rst=off  en=on  dbl_ovrd=off  block_type=register
  S-R Latch1 : Subsystem
  Ready : Outport
  Bit A : Outport  Port=2
  Bit B : Outport  Port=3
  Bits Valid : Outport  Port=4
  wires:
  IQ Valid/1 -> S-R Latch1/1(S), Register1/2, Logical2/1, Delay2/1
  S-R Latch1/1(Q) -> Inverter/1, Mux/1, Logical1/2
  Inverter/1 -> Ready/1
  Q/1 -> Register1/1
  Constant/1 -> AddSub/1
  Absolute/1 -> AddSub/2
  Mux/1 -> Bit A/1, Absolute/1
  I/1 -> Mux/2
  Register1/1 -> Mux/3
  AddSub/1 -> Bit B/1
  Delay2/1 -> Logical2/2
  Logical2/1 -> Bits Valid/1
  Logical1/1 -> S-R Latch1/2(R)
  Reest/1 -> Logical1/1

== system wlan_phy_rx_pmd/Detect & Decode/Soft Demap/64QAM Soft De-Map ==
  note: 64-QAM Demap:
      | Each sym is [b0 b1 b2 b3 b4 b5]
      | b0 = 1 if (I > 0)
      | b1 = 1 if(abs(I) < 4/sqrt(42))
      |
      | b2 = 1 if(2/sqrt(42) < abs(I) < 6/sqrt(42))
      | b3 = 1 if(Q > 0)
      |
      | b4 = 1 if(abs(Q) < 4/sqrt(42))
      | b5 = 1 if(2/sqrt(42) < abs(Q) < 6/sqrt(42))
  IQ Valid : Inport
  I : Inport  Port=2
  Q : Inport  Port=3
  Reest : Inport  Port=4
  64QAM Demap : Scope  NumInputPorts=8
      settings: TimeRange=20000  YMin=0~-1~0~0~-1~0~-1~-1  YMax=1~1~1~1~1~1~1~1  SaveName=ScopeData26
  Ctrl : Subsystem
  Gateway Out{1..6} (6 alike) : xbsIndex_r4/Gateway Out  inherit_from_input=off  hdl_port=off  timing_constraint=None  locs_specified=off  LOCs={}  UseAsDAC=off  DACChannel='1'  block_type=gatewayout
  Logical3 : xbsIndex_r4/Logical  logical_function=AND  inputs=2  en=off  latency=0  precision=Full  arith_type=Unsigned  n_bits=16  bin_pt=0  align_bp=on  dbl_ovrd=off  block_type=logical
  Mux : xbsIndex_r4/Mux  inputs=3  en=off  latency=0  precision=Full  arith_type=Unsigned  n_bits=16  bin_pt=14  quantization=Truncate  overflow=Wrap  dbl_ovrd=off  block_type=mux
  Mux1 : xbsIndex_r4/Mux  inputs=3  en=off  latency=0  precision=Full  arith_type=Unsigned  n_bits=16  bin_pt=14  quantization=Truncate  overflow=Wrap  dbl_ovrd=off  block_type=mux
  Register2 : xbsIndex_r4/Register  init=0  rst=off  en=on  dbl_ovrd=off  block_type=register
  Register3 : xbsIndex_r4/Register  init=0  rst=off  en=on  dbl_ovrd=off  block_type=register
  b1/b4 map : Subsystem
  b1/b4 map1 : Subsystem
  b2/b5 map : Subsystem
  b2/b5 map1 : Subsystem
  Ready : Outport
  Bit A : Outport  Port=2
  Bit B : Outport  Port=3
  Bits Valid : Outport  Port=4
  wires:
  I/1 -> Register2/1, Gateway Out3/1
  Mux/1 -> Bit A/1
  b1/b4 map/1(b) -> Mux1/2
  Logical3/1 -> Register2/2, Register3/2
  IQ Valid/1 -> Logical3/2, Ctrl/1(Vin), Gateway Out1/1
  b2/b5 map/1(b) -> Mux/3
  Register2/1 -> b2/b5 map/1(IQ), Mux/2, b1/b4 map/1(IQ)
  Register3/1 -> Mux1/3, b1/b4 map1/1(IQ), b2/b5 map1/1(IQ)
  Q/1 -> Register3/1, Gateway Out2/1
  b1/b4 map1/1(b) -> Mux/4
  b2/b5 map1/1(b) -> Mux1/4
  Mux1/1 -> Bit B/1
  Ctrl/3(Sel) -> Mux/1, Mux1/1, Gateway Out4/1
  Ctrl/2(Vout) -> Bits Valid/1, Gateway Out5/1
  Ctrl/1(Ready) -> Logical3/1, Ready/1, Gateway Out6/1
  Gateway Out1/1 -> 64QAM Demap/1  "valid in"
  Gateway Out3/1 -> 64QAM Demap/2  "I In"
  Gateway Out2/1 -> 64QAM Demap/3  "Q In"
  Gateway Out4/1 -> 64QAM Demap/4  "Mux Sel"
  Gateway Out5/1 -> 64QAM Demap/5  "Vout"
  Gateway Out6/1 -> 64QAM Demap/6  "Ready"
  Reest/1 -> Ctrl/2(Reest)

== system wlan_phy_rx_pmd/Detect & Decode/Soft Demap/BPSK Soft De-Map ==
  V_in : Inport
  I : Inport  Port=2
  Q : Inport  Port=3
  Reest : Inport  Port=4
  Constant : xbsIndex_r4/Constant  const=1  gui_display_data_type=Boolean  arith_type=Boolean  n_bits=16  bin_pt=14  preci_type=Single  exp_width=8  frac_width=24  explicit_period=on  period=1  equ=P=C  opselect=C  inp2=PCIN>>17  opr=+  inp1=P  carry=CIN  dbl_ovrd=off  block_type=constant
  Logical1 : xbsIndex_r4/Logical  logical_function=OR  inputs=2  en=off  latency=0  precision=Full  arith_type=Unsigned  n_bits=16  bin_pt=0  align_bp=on  dbl_ovrd=off  block_type=logical
  Logical2 : xbsIndex_r4/Logical  logical_function=AND  inputs=2  en=off  latency=0  precision=Full  arith_type=Unsigned  n_bits=16  bin_pt=0  align_bp=on  dbl_ovrd=off  block_type=logical
  Register2 : xbsIndex_r4/Register  init=0  rst=off  en=on  dbl_ovrd=off  block_type=register
  S-R Latch1 : Subsystem
  Ready : Outport
  Bit A : Outport  Port=2
  Bit B : Outport  Port=3
  Bits Valid : Outport  Port=4
  wires:
  I/1 -> Register2/1, Bit B/1
  Constant/1 -> Ready/1
  V_in/1 -> S-R Latch1/1(S), Register2/2, Logical2/2
  S-R Latch1/1(Q) -> Logical2/1
  Register2/1 -> Bit A/1
  Logical2/1 -> Bits Valid/1, Logical1/2
  Logical1/1 -> S-R Latch1/2(R)
  Reest/1 -> Logical1/1

== system wlan_phy_rx_pmd/Detect & Decode/Soft Demap/QPSK Soft De-Map ==
  IQ Valid : Inport
  I : Inport  Port=2
  Q : Inport  Port=3
  Reest : Inport  Port=4
  Constant1 : xbsIndex_r4/Constant  const=1  gui_display_data_type=Fixed-point  arith_type=Boolean  n_bits=1  bin_pt=0  preci_type=Single  exp_width=8  frac_width=24  explicit_period=off  period=1  equ=P=C  opselect=C  inp2=PCIN>>17  opr=+  inp1=P  carry=CIN  dbl_ovrd=off  block_type=constant
      settings: block_version=VER_STRING_GOES_HERE
  Ready : Outport
  Bit A : Outport  Port=2
  Bit B : Outport  Port=3
  Bits Valid : Outport  Port=4
  wires:
  I/1 -> Bit A/1
  Q/1 -> Bit B/1
  IQ Valid/1 -> Bits Valid/1
  Constant1/1 -> Ready/1
  Reest/1 -> ?

== system wlan_phy_rx_pmd/Detect & Decode/Soft Demap/Scale & Quantize ==
  Bit A : Inport
  Bit B : Inport  Port=2
  Bits Valid : Inport  Port=3
  mod_sel : Inport  Port=4
  Delay : xbsIndex_r4/Delay  rst=off  infoeditControl=Selection of Reset will increase slice count due to use of real FFs and instead of SRLs  en=off  latency=1  dbl_ovrd=off  reg_retiming=off  block_type=delay
  Delay1 : xbsIndex_r4/Delay  rst=off  infoeditControl=Selection of Reset will increase slice count due to use of real FFs and instead of SRLs  en=off  latency=1  dbl_ovrd=off  reg_retiming=off  block_type=delay
  Display : Display
  Display1 : Display
  Display2 : Display
  Gateway Out{1..8} (8 alike) : xbsIndex_r4/Gateway Out  inherit_from_input=off  hdl_port=off  timing_constraint=None  locs_specified=off  LOCs={}  UseAsDAC=off  DACChannel='1'  block_type=gatewayout
  Mult1 : xbsIndex_r4/Mult  precision=User Defined  arith_type="Signed  (2's comp)"  n_bits=13  bin_pt=0  quantization=Truncate  overflow=Saturate  en=off  latency=1  dbl_ovrd=off  use_behavioral_HDL=off  opt=Speed  use_embedded=on  optimum_pipeline=off  pipeline=on  use_rpm=on  placement_style=Triangular  block_type=mult
  Mult2 : xbsIndex_r4/Mult  precision=User Defined  arith_type="Signed  (2's comp)"  n_bits=13  bin_pt=0  quantization=Truncate  overflow=Saturate  en=off  latency=1  dbl_ovrd=off  use_behavioral_HDL=off  opt=Speed  use_embedded=on  optimum_pipeline=off  pipeline=on  use_rpm=on  placement_style=Triangular  block_type=mult
  Mux : xbsIndex_r4/Mux  inputs=4  en=off  latency=0  precision=Full  arith_type=Unsigned  n_bits=16  bin_pt=14  quantization=Truncate  overflow=Wrap  dbl_ovrd=off  block_type=mux
  Negate : xbsIndex_r4/Negate  precision=User Defined  arith_type="Signed  (2's comp)"  n_bits=4  bin_pt=0  quantization=Truncate  overflow=Saturate  en=off  latency=0  dbl_ovrd=off  block_type=negate
  Negate1 : xbsIndex_r4/Negate  precision=User Defined  arith_type="Signed  (2's comp)"  n_bits=4  bin_pt=0  quantization=Truncate  overflow=Saturate  en=off  latency=0  dbl_ovrd=off  block_type=negate
  Quantize : Subsystem
  Quantize1 : Subsystem
  Reinterpret : xbsIndex_r4/Reinterpret  force_arith_type=off  arith_type="Signed  (2's comp)"  force_bin_pt=on  bin_pt=0  block_type=reinterpret
  Reinterpret1 : xbsIndex_r4/Reinterpret  force_arith_type=off  arith_type="Signed  (2's comp)"  force_bin_pt=on  bin_pt=0  block_type=reinterpret
  Scale/Quant : Scope  NumInputPorts=8
      settings: TimeRange=178.0593691607082  YMin=-1~-95.55~5.7~-573.3~-4.2~-1~-1~-1  YMax=1~-86.45~6.3~-518.7~-3.8~1~1~1  SaveName=ScopeData2
  LLR A : Outport
  LLR B : Outport  Port=2
  LLRs Valid : Outport  Port=3
  " mod_sel" : Outport  Port=4
  wires:
  mod_sel/1 -> Mux/1, Delay1/1
  [regRx_FEC_SCALE_QPSK] -> Mux/3, Mux/2, Gateway Out6/1
  [regRx_FEC_SCALE_16QAM] -> Mux/4, Gateway Out7/1
  [regRx_FEC_SCALE_64QAM] -> Mux/5, Gateway Out8/1
  Bit A/1 -> Reinterpret/1
  Mult1/1 -> Quantize/1(D), Gateway Out1/1
  Quantize/1(Q) -> Gateway Out5/1, Negate/1
  Mux/1 -> Mult1/2, Gateway Out4/1, Mult2/2
  Bits Valid/1 -> Delay/1, Gateway Out2/1
  Delay/1 -> LLRs Valid/1
  Delay1/1 -> " mod_sel"/1
  Gateway Out2/1 -> Scale/Quant/1  "In Valid"
  Gateway Out3/1 -> Scale/Quant/2  "Soft Bit In"
  Gateway Out4/1 -> Scale/Quant/3  "Scale"
  Gateway Out1/1 -> Scale/Quant/4  "Bit Scaled"
  Gateway Out5/1 -> Scale/Quant/5  "LLR"
  Reinterpret/1 -> Mult1/1, Gateway Out3/1
  Negate/1 -> LLR A/1
  Gateway Out8/1 -> Display2/1
  Gateway Out7/1 -> Display1/1
  Gateway Out6/1 -> Display/1
  Bit B/1 -> Reinterpret1/1
  Reinterpret1/1 -> Mult2/1
  Mult2/1 -> Quantize1/1(D)
  Quantize1/1(Q) -> Negate1/1
  Negate1/1 -> LLR B/1

== system wlan_phy_rx_pmd/Detect & Decode/Sym FIFO/S-R Latch1 ==
  S : Inport
  R : Inport  Port=2
  Constant1 : xbsIndex_r4/Constant  const=1  gui_display_data_type=Fixed-point  arith_type=Boolean  n_bits=1  bin_pt=0  preci_type=Single  exp_width=8  frac_width=24  explicit_period=off  period=1  equ=P=C  opselect=C  inp2=PCIN>>17  opr=+  inp1=P  carry=CIN  dbl_ovrd=off  block_type=constant
      settings: block_version=VER_STRING_GOES_HERE
  Convert1 : xbsIndex_r4/Convert  gui_display_data_type=Boolean  arith_type=Boolean  n_bits=1  bin_pt=0  float_type=Single  exp_bits=8  fraction_bits=24  quantization=Truncate  overflow=Wrap  en=off  latency=0  dbl_ovrd=off  pipeline=off  block_type=convert
  Convert2 : xbsIndex_r4/Convert  gui_display_data_type=Boolean  arith_type=Boolean  n_bits=1  bin_pt=0  float_type=Single  exp_bits=8  fraction_bits=24  quantization=Truncate  overflow=Wrap  en=off  latency=0  dbl_ovrd=off  pipeline=off  block_type=convert
  Register2 : xbsIndex_r4/Register  init=0  rst=on  en=on  dbl_ovrd=off  block_type=register
      settings: xl_area=[1 1 0 0 0 0 0]  block_version=VER_STRING_GOES_HERE
  Q : Outport
  wires:
  S/1 -> Convert2/1
  Register2/1 -> Q/1
  R/1 -> Convert1/1
  Constant1/1 -> Register2/1
  Convert1/1 -> Register2/2
  Convert2/1 -> Register2/3
